simulink library "Garbage"  (R2023a)
settings: Solver=ode45  StartTime=0.0  StopTime=10.0  RelTol=1e-3  EnableMultiTasking=on
undefined symbols (not defined in the file or in its folder): c, ending_column_index, ending_row_index, framebuffer_16x1, framebuffer_2x2, framebuffer_4x4, framebuffer_8x8, HEIGHT, hot, POS_X, POS_Y, starting_column_index, starting_row_index, WIDTH
libraries: Memory_Blocks_Lib x459, simulink x235, simulink_extras x132, dspmtrx3 x116, Bit_Multiplexers_Lib x67, Bit_Demultiplexers_Lib x49, Byte_Multiplexers_Lib x40, Miscellaneous x38, Computer_Library x17, Decoders_Lib x14, commutil2 x12, Arithmetic_Operations_Lib x8
(part 5 of 26)

== system Garbage/Byte_Multiplexer_2_to_1 ==
  Addr(1b) : Inport
  Data_1(1B) : Inport  Port=2
  Data_0(1B) : Inport  Port=3
  Bit_Multiplexer_2_to_1(Bit_0) : Bit_Multiplexers_Lib/Bit_Multiplexers_Lib/Bit_Multiplexer_2_to_1  SystemSampleTime=-1  VariantActivationTime=update diagram  Latency=0  AutoFrameSizeCalculation=off
  Bit_Multiplexer_2_to_1(Bit_1) : Bit_Multiplexers_Lib/Bit_Multiplexers_Lib/Bit_Multiplexer_2_to_1  SystemSampleTime=-1  VariantActivationTime=update diagram  Latency=0  AutoFrameSizeCalculation=off
  Bit_Multiplexer_2_to_1(Bit_2) : Bit_Multiplexers_Lib/Bit_Multiplexers_Lib/Bit_Multiplexer_2_to_1  SystemSampleTime=-1  VariantActivationTime=update diagram  Latency=0  AutoFrameSizeCalculation=off
  Bit_Multiplexer_2_to_1(Bit_3) : Bit_Multiplexers_Lib/Bit_Multiplexers_Lib/Bit_Multiplexer_2_to_1  SystemSampleTime=-1  VariantActivationTime=update diagram  Latency=0  AutoFrameSizeCalculation=off
  Bit_Multiplexer_2_to_1(Bit_4) : Bit_Multiplexers_Lib/Bit_Multiplexers_Lib/Bit_Multiplexer_2_to_1  SystemSampleTime=-1  VariantActivationTime=update diagram  Latency=0  AutoFrameSizeCalculation=off
  Bit_Multiplexer_2_to_1(Bit_5) : Bit_Multiplexers_Lib/Bit_Multiplexers_Lib/Bit_Multiplexer_2_to_1  SystemSampleTime=-1  VariantActivationTime=update diagram  Latency=0  AutoFrameSizeCalculation=off
  Bit_Multiplexer_2_to_1(Bit_6) : Bit_Multiplexers_Lib/Bit_Multiplexers_Lib/Bit_Multiplexer_2_to_1  SystemSampleTime=-1  VariantActivationTime=update diagram  Latency=0  AutoFrameSizeCalculation=off
  Bit_Multiplexer_2_to_1(Bit_7) : Bit_Multiplexers_Lib/Bit_Multiplexers_Lib/Bit_Multiplexer_2_to_1  SystemSampleTime=-1  VariantActivationTime=update diagram  Latency=0  AutoFrameSizeCalculation=off
  Bus Creator : BusCreator  Inputs=8
  Bus Selector : BusSelector  OutputSignals=signal1,signal2,signal3,signal4,signal5,signal6,signal7,signal8
  Bus Selector1 : BusSelector  OutputSignals=signal1,signal2,signal3,signal4,signal5,signal6,signal7,signal8
  Data(1B) : Outport
  wires:
  Bus Creator/1 -> Data(1B)/1
  Addr(1b)/1 -> Bit_Multiplexer_2_to_1(Bit_7)/1, Bit_Multiplexer_2_to_1(Bit_0)/1, Bit_Multiplexer_2_to_1(Bit_1)/1, Bit_Multiplexer_2_to_1(Bit_2)/1, Bit_Multiplexer_2_to_1(Bit_3)/1, Bit_Multiplexer_2_to_1(Bit_4)/1, Bit_Multiplexer_2_to_1(Bit_5)/1, Bit_Multiplexer_2_to_1(Bit_6)/1
  Bit_Multiplexer_2_to_1(Bit_4)/1 -> Bus Creator/4
  Bit_Multiplexer_2_to_1(Bit_5)/1 -> Bus Creator/3
  Bit_Multiplexer_2_to_1(Bit_6)/1 -> Bus Creator/2
  Bit_Multiplexer_2_to_1(Bit_7)/1 -> Bus Creator/1
  Bit_Multiplexer_2_to_1(Bit_3)/1 -> Bus Creator/5
  Bit_Multiplexer_2_to_1(Bit_2)/1 -> Bus Creator/6
  Bit_Multiplexer_2_to_1(Bit_1)/1 -> Bus Creator/7
  Bit_Multiplexer_2_to_1(Bit_0)/1 -> Bus Creator/8
  Data_1(1B)/1 -> Bus Selector1/1
  Data_0(1B)/1 -> Bus Selector/1
  Bus Selector1/8 -> Bit_Multiplexer_2_to_1(Bit_0)/2  "<signal8>"
  Bus Selector/8 -> Bit_Multiplexer_2_to_1(Bit_0)/3  "<signal8>"
  Bus Selector1/7 -> Bit_Multiplexer_2_to_1(Bit_1)/2  "<signal7>"
  Bus Selector/7 -> Bit_Multiplexer_2_to_1(Bit_1)/3  "<signal7>"
  Bus Selector1/6 -> Bit_Multiplexer_2_to_1(Bit_2)/2  "<signal6>"
  Bus Selector/6 -> Bit_Multiplexer_2_to_1(Bit_2)/3  "<signal6>"
  Bus Selector/5 -> Bit_Multiplexer_2_to_1(Bit_3)/3  "<signal5>"
  Bus Selector1/5 -> Bit_Multiplexer_2_to_1(Bit_3)/2  "<signal5>"
  Bus Selector/4 -> Bit_Multiplexer_2_to_1(Bit_4)/3  "<signal4>"
  Bus Selector1/4 -> Bit_Multiplexer_2_to_1(Bit_4)/2  "<signal4>"
  Bus Selector1/3 -> Bit_Multiplexer_2_to_1(Bit_5)/2  "<signal3>"
  Bus Selector/3 -> Bit_Multiplexer_2_to_1(Bit_5)/3  "<signal3>"
  Bus Selector1/2 -> Bit_Multiplexer_2_to_1(Bit_6)/2  "<signal2>"
  Bus Selector/2 -> Bit_Multiplexer_2_to_1(Bit_6)/3  "<signal2>"
  Bus Selector/1 -> Bit_Multiplexer_2_to_1(Bit_7)/3  "<signal1>"
  Bus Selector1/1 -> Bit_Multiplexer_2_to_1(Bit_7)/2  "<signal1>"

== system Garbage/Byte_Multiplexer_4_to_1 ==
  Addr_1(1b) : Inport
  Addr_0(1b) : Inport  Port=2
  Data_3(1B) : Inport  Port=3
  Data_2(1B) : Inport  Port=4
  Data_1(1B) : Inport  Port=5
  Data_0(1B) : Inport  Port=6
  Bit_Multiplexer_4_to_1(Bit_0) : Bit_Multiplexers_Lib/Bit_Mux_4  SystemSampleTime=-1  VariantActivationTime=update diagram  Latency=0  AutoFrameSizeCalculation=off
  Bit_Multiplexer_4_to_1(Bit_1) : Bit_Multiplexers_Lib/Bit_Mux_4  SystemSampleTime=-1  VariantActivationTime=update diagram  Latency=0  AutoFrameSizeCalculation=off
  Bit_Multiplexer_4_to_1(Bit_2) : Bit_Multiplexers_Lib/Bit_Mux_4  SystemSampleTime=-1  VariantActivationTime=update diagram  Latency=0  AutoFrameSizeCalculation=off
  Bit_Multiplexer_4_to_1(Bit_3) : Bit_Multiplexers_Lib/Bit_Mux_4  SystemSampleTime=-1  VariantActivationTime=update diagram  Latency=0  AutoFrameSizeCalculation=off
  Bit_Multiplexer_4_to_1(Bit_4) : Bit_Multiplexers_Lib/Bit_Mux_4  SystemSampleTime=-1  VariantActivationTime=update diagram  Latency=0  AutoFrameSizeCalculation=off
  Bit_Multiplexer_4_to_1(Bit_5) : Bit_Multiplexers_Lib/Bit_Mux_4  SystemSampleTime=-1  VariantActivationTime=update diagram  Latency=0  AutoFrameSizeCalculation=off
  Bit_Multiplexer_4_to_1(Bit_6) : Bit_Multiplexers_Lib/Bit_Mux_4  SystemSampleTime=-1  VariantActivationTime=update diagram  Latency=0  AutoFrameSizeCalculation=off
  Bit_Multiplexer_4_to_1(Bit_7) : Bit_Multiplexers_Lib/Bit_Mux_4  SystemSampleTime=-1  VariantActivationTime=update diagram  Latency=0  AutoFrameSizeCalculation=off
  Bus Creator3 : BusCreator  Inputs=8
  Bus Selector{,1..3} (4 alike) : BusSelector  OutputSignals=signal1,signal2,signal3,signal4,signal5,signal6,signal7,signal8
  Data(1B) : Outport
  wires:
  Bus Creator3/1 -> Data(1B)/1
  Addr_0(1b)/1 -> Bit_Multiplexer_4_to_1(Bit_0)/2, Bit_Multiplexer_4_to_1(Bit_1)/2, Bit_Multiplexer_4_to_1(Bit_2)/2, Bit_Multiplexer_4_to_1(Bit_3)/2, Bit_Multiplexer_4_to_1(Bit_4)/2, Bit_Multiplexer_4_to_1(Bit_5)/2, Bit_Multiplexer_4_to_1(Bit_6)/2, Bit_Multiplexer_4_to_1(Bit_7)/2
  Addr_1(1b)/1 -> Bit_Multiplexer_4_to_1(Bit_7)/1, Bit_Multiplexer_4_to_1(Bit_0)/1, Bit_Multiplexer_4_to_1(Bit_1)/1, Bit_Multiplexer_4_to_1(Bit_2)/1, Bit_Multiplexer_4_to_1(Bit_3)/1, Bit_Multiplexer_4_to_1(Bit_4)/1, Bit_Multiplexer_4_to_1(Bit_5)/1, Bit_Multiplexer_4_to_1(Bit_6)/1
  Bit_Multiplexer_4_to_1(Bit_4)/1 -> Bus Creator3/4
  Bit_Multiplexer_4_to_1(Bit_5)/1 -> Bus Creator3/3
  Bit_Multiplexer_4_to_1(Bit_6)/1 -> Bus Creator3/2
  Bit_Multiplexer_4_to_1(Bit_7)/1 -> Bus Creator3/1
  Bit_Multiplexer_4_to_1(Bit_3)/1 -> Bus Creator3/5
  Bit_Multiplexer_4_to_1(Bit_2)/1 -> Bus Creator3/6
  Bit_Multiplexer_4_to_1(Bit_1)/1 -> Bus Creator3/7
  Bit_Multiplexer_4_to_1(Bit_0)/1 -> Bus Creator3/8
  Data_3(1B)/1 -> Bus Selector/1
  Data_2(1B)/1 -> Bus Selector1/1
  Data_1(1B)/1 -> Bus Selector2/1
  Data_0(1B)/1 -> Bus Selector3/1
  Bus Selector/8 -> Bit_Multiplexer_4_to_1(Bit_0)/3  "<signal8>"
  Bus Selector1/8 -> Bit_Multiplexer_4_to_1(Bit_0)/4  "<signal8>"
  Bus Selector2/8 -> Bit_Multiplexer_4_to_1(Bit_0)/5  "<signal8>"
  Bus Selector3/8 -> Bit_Multiplexer_4_to_1(Bit_0)/6  "<signal8>"
  Bus Selector/7 -> Bit_Multiplexer_4_to_1(Bit_1)/3  "<signal7>"
  Bus Selector1/7 -> Bit_Multiplexer_4_to_1(Bit_1)/4  "<signal7>"
  Bus Selector2/7 -> Bit_Multiplexer_4_to_1(Bit_1)/5  "<signal7>"
  Bus Selector3/7 -> Bit_Multiplexer_4_to_1(Bit_1)/6  "<signal7>"
  Bus Selector/6 -> Bit_Multiplexer_4_to_1(Bit_2)/3  "<signal6>"
  Bus Selector1/6 -> Bit_Multiplexer_4_to_1(Bit_2)/4  "<signal6>"
  Bus Selector2/6 -> Bit_Multiplexer_4_to_1(Bit_2)/5  "<signal6>"
  Bus Selector3/6 -> Bit_Multiplexer_4_to_1(Bit_2)/6  "<signal6>"
  Bus Selector/5 -> Bit_Multiplexer_4_to_1(Bit_3)/3  "<signal5>"
  Bus Selector1/5 -> Bit_Multiplexer_4_to_1(Bit_3)/4  "<signal5>"
  Bus Selector2/5 -> Bit_Multiplexer_4_to_1(Bit_3)/5  "<signal5>"
  Bus Selector3/5 -> Bit_Multiplexer_4_to_1(Bit_3)/6  "<signal5>"
  Bus Selector/4 -> Bit_Multiplexer_4_to_1(Bit_4)/3  "<signal4>"
  Bus Selector1/4 -> Bit_Multiplexer_4_to_1(Bit_4)/4  "<signal4>"
  Bus Selector2/4 -> Bit_Multiplexer_4_to_1(Bit_4)/5  "<signal4>"
  Bus Selector3/4 -> Bit_Multiplexer_4_to_1(Bit_4)/6  "<signal4>"
  Bus Selector/3 -> Bit_Multiplexer_4_to_1(Bit_5)/3  "<signal3>"
  Bus Selector1/3 -> Bit_Multiplexer_4_to_1(Bit_5)/4  "<signal3>"
  Bus Selector2/3 -> Bit_Multiplexer_4_to_1(Bit_5)/5  "<signal3>"
  Bus Selector3/3 -> Bit_Multiplexer_4_to_1(Bit_5)/6  "<signal3>"
  Bus Selector/2 -> Bit_Multiplexer_4_to_1(Bit_6)/3  "<signal2>"
  Bus Selector1/2 -> Bit_Multiplexer_4_to_1(Bit_6)/4  "<signal2>"
  Bus Selector2/2 -> Bit_Multiplexer_4_to_1(Bit_6)/5  "<signal2>"
  Bus Selector3/2 -> Bit_Multiplexer_4_to_1(Bit_6)/6  "<signal2>"
  Bus Selector/1 -> Bit_Multiplexer_4_to_1(Bit_7)/3  "<signal1>"
  Bus Selector1/1 -> Bit_Multiplexer_4_to_1(Bit_7)/4  "<signal1>"
  Bus Selector2/1 -> Bit_Multiplexer_4_to_1(Bit_7)/5  "<signal1>"
  Bus Selector3/1 -> Bit_Multiplexer_4_to_1(Bit_7)/6  "<signal1>"

== system Garbage/Byte_Multiplexer_8_to_1 ==
  Addr_2(1b) : Inport
  Addr_1(1b) : Inport  Port=2
  Addr_0(1b) : Inport  Port=3
  Data_7(1B) : Inport  Port=4
  Data_6(1B) : Inport  Port=5
  Data_5(1B) : Inport  Port=6
  Data_4(1B) : Inport  Port=7
  Data_3(1B) : Inport  Port=8
  Data_2(1B) : Inport  Port=9
  Data_1(1B) : Inport  Port=10
  Data_0(1B) : Inport  Port=11
  Bit_Multiplexer_8_to_1(Bit_0) : Bit_Multiplexers_Lib/Bit_MltPlx_8  SystemSampleTime=-1  VariantActivationTime=update diagram  Latency=0  AutoFrameSizeCalculation=off
  Bit_Multiplexer_8_to_1(Bit_1) : Bit_Multiplexers_Lib/Bit_MltPlx_8  SystemSampleTime=-1  VariantActivationTime=update diagram  Latency=0  AutoFrameSizeCalculation=off
  Bit_Multiplexer_8_to_1(Bit_2) : Bit_Multiplexers_Lib/Bit_MltPlx_8  SystemSampleTime=-1  VariantActivationTime=update diagram  Latency=0  AutoFrameSizeCalculation=off
  Bit_Multiplexer_8_to_1(Bit_3) : Bit_Multiplexers_Lib/Bit_MltPlx_8  SystemSampleTime=-1  VariantActivationTime=update diagram  Latency=0  AutoFrameSizeCalculation=off
  Bit_Multiplexer_8_to_1(Bit_4) : Bit_Multiplexers_Lib/Bit_MltPlx_8  SystemSampleTime=-1  VariantActivationTime=update diagram  Latency=0  AutoFrameSizeCalculation=off
  Bit_Multiplexer_8_to_1(Bit_5) : Bit_Multiplexers_Lib/Bit_MltPlx_8  SystemSampleTime=-1  VariantActivationTime=update diagram  Latency=0  AutoFrameSizeCalculation=off
  Bit_Multiplexer_8_to_1(Bit_6) : Bit_Multiplexers_Lib/Bit_MltPlx_8  SystemSampleTime=-1  VariantActivationTime=update diagram  Latency=0  AutoFrameSizeCalculation=off
  Bit_Multiplexer_8_to_1(Bit_7) : Bit_Multiplexers_Lib/Bit_MltPlx_8  SystemSampleTime=-1  VariantActivationTime=update diagram  Latency=0  AutoFrameSizeCalculation=off
  Bus Creator3 : BusCreator  Inputs=8
  Bus Selector{,1..7} (8 alike) : BusSelector  OutputSignals=signal1,signal2,signal3,signal4,signal5,signal6,signal7,signal8
  Data(1B) : Outport
  wires:
  Bus Creator3/1 -> Data(1B)/1
  Addr_0(1b)/1 -> Bit_Multiplexer_8_to_1(Bit_0)/3, Bit_Multiplexer_8_to_1(Bit_1)/3, Bit_Multiplexer_8_to_1(Bit_2)/3, Bit_Multiplexer_8_to_1(Bit_3)/3, Bit_Multiplexer_8_to_1(Bit_4)/3, Bit_Multiplexer_8_to_1(Bit_5)/3, Bit_Multiplexer_8_to_1(Bit_6)/3, Bit_Multiplexer_8_to_1(Bit_7)/3
  Addr_1(1b)/1 -> Bit_Multiplexer_8_to_1(Bit_5)/2, Bit_Multiplexer_8_to_1(Bit_0)/2, Bit_Multiplexer_8_to_1(Bit_1)/2, Bit_Multiplexer_8_to_1(Bit_2)/2, Bit_Multiplexer_8_to_1(Bit_3)/2, Bit_Multiplexer_8_to_1(Bit_4)/2, Bit_Multiplexer_8_to_1(Bit_6)/2, Bit_Multiplexer_8_to_1(Bit_7)/2
  Addr_2(1b)/1 -> Bit_Multiplexer_8_to_1(Bit_0)/1, Bit_Multiplexer_8_to_1(Bit_1)/1, Bit_Multiplexer_8_to_1(Bit_2)/1, Bit_Multiplexer_8_to_1(Bit_3)/1, Bit_Multiplexer_8_to_1(Bit_4)/1, Bit_Multiplexer_8_to_1(Bit_5)/1, Bit_Multiplexer_8_to_1(Bit_6)/1, Bit_Multiplexer_8_to_1(Bit_7)/1
  Bit_Multiplexer_8_to_1(Bit_4)/1 -> Bus Creator3/4
  Bit_Multiplexer_8_to_1(Bit_5)/1 -> Bus Creator3/3
  Bit_Multiplexer_8_to_1(Bit_6)/1 -> Bus Creator3/2
  Bit_Multiplexer_8_to_1(Bit_7)/1 -> Bus Creator3/1
  Bit_Multiplexer_8_to_1(Bit_3)/1 -> Bus Creator3/5
  Bit_Multiplexer_8_to_1(Bit_2)/1 -> Bus Creator3/6
  Bit_Multiplexer_8_to_1(Bit_1)/1 -> Bus Creator3/7
  Bit_Multiplexer_8_to_1(Bit_0)/1 -> Bus Creator3/8
  Data_7(1B)/1 -> Bus Selector/1
  Data_6(1B)/1 -> Bus Selector1/1
  Data_5(1B)/1 -> Bus Selector2/1
  Data_4(1B)/1 -> Bus Selector3/1
  Data_3(1B)/1 -> Bus Selector4/1
  Data_2(1B)/1 -> Bus Selector5/1
  Data_1(1B)/1 -> Bus Selector6/1
  Data_0(1B)/1 -> Bus Selector7/1
  Bus Selector/1 -> Bit_Multiplexer_8_to_1(Bit_7)/4  "<signal1>"
  Bus Selector1/1 -> Bit_Multiplexer_8_to_1(Bit_7)/5  "<signal1>"
  Bus Selector2/1 -> Bit_Multiplexer_8_to_1(Bit_7)/6  "<signal1>"
  Bus Selector3/1 -> Bit_Multiplexer_8_to_1(Bit_7)/7  "<signal1>"
  Bus Selector4/1 -> Bit_Multiplexer_8_to_1(Bit_7)/8  "<signal1>"
  Bus Selector5/1 -> Bit_Multiplexer_8_to_1(Bit_7)/9  "<signal1>"
  Bus Selector6/1 -> Bit_Multiplexer_8_to_1(Bit_7)/10  "<signal1>"
  Bus Selector7/1 -> Bit_Multiplexer_8_to_1(Bit_7)/11  "<signal1>"
  Bus Selector/2 -> Bit_Multiplexer_8_to_1(Bit_6)/4  "<signal2>"
  Bus Selector1/2 -> Bit_Multiplexer_8_to_1(Bit_6)/5  "<signal2>"
  Bus Selector2/2 -> Bit_Multiplexer_8_to_1(Bit_6)/6  "<signal2>"
  Bus Selector3/2 -> Bit_Multiplexer_8_to_1(Bit_6)/7  "<signal2>"
  Bus Selector4/2 -> Bit_Multiplexer_8_to_1(Bit_6)/8  "<signal2>"
  Bus Selector5/2 -> Bit_Multiplexer_8_to_1(Bit_6)/9  "<signal2>"
  Bus Selector6/2 -> Bit_Multiplexer_8_to_1(Bit_6)/10  "<signal2>"
  Bus Selector7/2 -> Bit_Multiplexer_8_to_1(Bit_6)/11  "<signal2>"
  Bus Selector/3 -> Bit_Multiplexer_8_to_1(Bit_5)/4  "<signal3>"
  Bus Selector1/3 -> Bit_Multiplexer_8_to_1(Bit_5)/5  "<signal3>"
  Bus Selector2/3 -> Bit_Multiplexer_8_to_1(Bit_5)/6  "<signal3>"
  Bus Selector3/3 -> Bit_Multiplexer_8_to_1(Bit_5)/7  "<signal3>"
  Bus Selector4/3 -> Bit_Multiplexer_8_to_1(Bit_5)/8  "<signal3>"
  Bus Selector5/3 -> Bit_Multiplexer_8_to_1(Bit_5)/9  "<signal3>"
  Bus Selector6/3 -> Bit_Multiplexer_8_to_1(Bit_5)/10  "<signal3>"
  Bus Selector7/3 -> Bit_Multiplexer_8_to_1(Bit_5)/11  "<signal3>"
  Bus Selector/4 -> Bit_Multiplexer_8_to_1(Bit_4)/4  "<signal4>"
  Bus Selector1/4 -> Bit_Multiplexer_8_to_1(Bit_4)/5  "<signal4>"
  Bus Selector2/4 -> Bit_Multiplexer_8_to_1(Bit_4)/6  "<signal4>"
  Bus Selector3/4 -> Bit_Multiplexer_8_to_1(Bit_4)/7  "<signal4>"
  Bus Selector4/4 -> Bit_Multiplexer_8_to_1(Bit_4)/8  "<signal4>"
  Bus Selector5/4 -> Bit_Multiplexer_8_to_1(Bit_4)/9  "<signal4>"
  Bus Selector6/4 -> Bit_Multiplexer_8_to_1(Bit_4)/10  "<signal4>"
  Bus Selector7/4 -> Bit_Multiplexer_8_to_1(Bit_4)/11  "<signal4>"
  Bus Selector/5 -> Bit_Multiplexer_8_to_1(Bit_3)/4  "<signal5>"
  Bus Selector1/5 -> Bit_Multiplexer_8_to_1(Bit_3)/5  "<signal5>"
  Bus Selector2/5 -> Bit_Multiplexer_8_to_1(Bit_3)/6  "<signal5>"
  Bus Selector3/5 -> Bit_Multiplexer_8_to_1(Bit_3)/7  "<signal5>"
  Bus Selector4/5 -> Bit_Multiplexer_8_to_1(Bit_3)/8  "<signal5>"
  Bus Selector5/5 -> Bit_Multiplexer_8_to_1(Bit_3)/9  "<signal5>"
  Bus Selector6/5 -> Bit_Multiplexer_8_to_1(Bit_3)/10  "<signal5>"
  Bus Selector7/5 -> Bit_Multiplexer_8_to_1(Bit_3)/11  "<signal5>"
  Bus Selector/6 -> Bit_Multiplexer_8_to_1(Bit_2)/4  "<signal6>"
  Bus Selector1/6 -> Bit_Multiplexer_8_to_1(Bit_2)/5  "<signal6>"
  Bus Selector2/6 -> Bit_Multiplexer_8_to_1(Bit_2)/6  "<signal6>"
  Bus Selector3/6 -> Bit_Multiplexer_8_to_1(Bit_2)/7  "<signal6>"
  Bus Selector4/6 -> Bit_Multiplexer_8_to_1(Bit_2)/8  "<signal6>"
  Bus Selector5/6 -> Bit_Multiplexer_8_to_1(Bit_2)/9  "<signal6>"
  Bus Selector6/6 -> Bit_Multiplexer_8_to_1(Bit_2)/10  "<signal6>"
  Bus Selector7/6 -> Bit_Multiplexer_8_to_1(Bit_2)/11  "<signal6>"
  Bus Selector/7 -> Bit_Multiplexer_8_to_1(Bit_1)/4  "<signal7>"
  Bus Selector1/7 -> Bit_Multiplexer_8_to_1(Bit_1)/5  "<signal7>"
  Bus Selector2/7 -> Bit_Multiplexer_8_to_1(Bit_1)/6  "<signal7>"
  Bus Selector3/7 -> Bit_Multiplexer_8_to_1(Bit_1)/7  "<signal7>"
  Bus Selector4/7 -> Bit_Multiplexer_8_to_1(Bit_1)/8  "<signal7>"
  Bus Selector5/7 -> Bit_Multiplexer_8_to_1(Bit_1)/9  "<signal7>"
  Bus Selector6/7 -> Bit_Multiplexer_8_to_1(Bit_1)/10  "<signal7>"
  Bus Selector7/7 -> Bit_Multiplexer_8_to_1(Bit_1)/11  "<signal7>"
  Bus Selector/8 -> Bit_Multiplexer_8_to_1(Bit_0)/4  "<signal8>"
  Bus Selector1/8 -> Bit_Multiplexer_8_to_1(Bit_0)/5  "<signal8>"
  Bus Selector2/8 -> Bit_Multiplexer_8_to_1(Bit_0)/6  "<signal8>"
  Bus Selector3/8 -> Bit_Multiplexer_8_to_1(Bit_0)/7  "<signal8>"
  Bus Selector4/8 -> Bit_Multiplexer_8_to_1(Bit_0)/8  "<signal8>"
  Bus Selector5/8 -> Bit_Multiplexer_8_to_1(Bit_0)/9  "<signal8>"
  Bus Selector6/8 -> Bit_Multiplexer_8_to_1(Bit_0)/10  "<signal8>"
  Bus Selector7/8 -> Bit_Multiplexer_8_to_1(Bit_0)/11  "<signal8>"

== system Garbage/Counter_15bit ==
  CLK : Inport
  Bit_00 : simulink_extras/Flip Flops/J-K Flip-Flop  initial_condition=0
  Bit_01 : simulink_extras/Flip Flops/J-K Flip-Flop  initial_condition=0
  Bit_02 : simulink_extras/Flip Flops/J-K Flip-Flop  initial_condition=0
  Bit_03 : simulink_extras/Flip Flops/J-K Flip-Flop  initial_condition=0
  Bit_04 : simulink_extras/Flip Flops/J-K Flip-Flop  initial_condition=0
  Bit_05 : simulink_extras/Flip Flops/J-K Flip-Flop  initial_condition=0
  Bit_06 : simulink_extras/Flip Flops/J-K Flip-Flop  initial_condition=0
  Bit_07 : simulink_extras/Flip Flops/J-K Flip-Flop  initial_condition=0
  Bit_08 : simulink_extras/Flip Flops/J-K Flip-Flop  initial_condition=0
  Bit_09 : simulink_extras/Flip Flops/J-K Flip-Flop  initial_condition=0
  Bit_{10..14} (5 alike) : simulink_extras/Flip Flops/J-K Flip-Flop  initial_condition=0
  Increment Enabled1 : Logic  IconShape=distinctive  AllPortsSameDT=off  OutDataTypeStr=boolean
  Increment Enabled2 : Logic  IconShape=distinctive  AllPortsSameDT=off  OutDataTypeStr=boolean
  Increment(1b) : Constant  OutDataTypeStr=boolean
  Logical Operator{1..10,13} (11 alike) : Logic  IconShape=distinctive  AllPortsSameDT=off  OutDataTypeStr=boolean
  Logical Operator11 : Logic  Inputs=15  IconShape=distinctive  AllPortsSameDT=off  OutDataTypeStr=boolean
  Terminator{1..15} (15 alike) : Terminator
  1Hz : Outport
  wires:
  Bit_00/2 -> Terminator9/1
  Increment(1b)/1 -> Bit_00/3, Bit_00/1
  Bit_02/2 -> Terminator11/1
  Bit_03/2 -> Terminator12/1
  Bit_01/2 -> Terminator10/1
  Logical Operator2/1 -> Logical Operator10/1, Bit_07/1, Bit_07/3
  Bit_07/1 -> Logical Operator11/8, Logical Operator10/2
  Bit_06/2 -> Terminator2/1
  Bit_05/2 -> Terminator1/1
  Increment Enabled1/1 -> Bit_05/3, Bit_05/1, Logical Operator1/1
  Bit_07/2 -> Terminator3/1
  Logical Operator13/1 -> Increment Enabled1/1, Bit_04/3, Bit_04/1
  Logical Operator1/1 -> Logical Operator2/1, Bit_06/1, Bit_06/3
  Logical Operator4/1 -> Logical Operator13/1, Bit_03/3, Bit_03/1
  Bit_04/2 -> Terminator4/1
  Logical Operator3/1 -> Logical Operator4/1, Bit_02/3, Bit_02/1
  Bit_09/1 -> Logical Operator11/10, Logical Operator9/2
  Logical Operator10/1 -> Bit_08/1, Bit_08/3, Logical Operator8/1
  Bit_10/1 -> Logical Operator11/11, Logical Operator6/2
  Bit_09/2 -> Terminator7/1
  Bit_10/2 -> Terminator8/1
  Bit_08/2 -> Terminator6/1
  Bit_08/1 -> Logical Operator11/9, Logical Operator8/2
  Bit_13/1 -> Logical Operator11/14, Logical Operator7/2
  Bit_11/1 -> Logical Operator11/12, Increment Enabled2/2
  Bit_12/1 -> Logical Operator11/13, Logical Operator5/2
  Logical Operator7/1 -> Bit_14/1, Bit_14/3
  CLK/1 -> Bit_00/2, Bit_01/2, Bit_02/2, Bit_03/2, Bit_04/2, Bit_05/2, Bit_06/2, Bit_07/2, Bit_08/2, Bit_09/2, Bit_10/2, Bit_11/2, Bit_12/2, Bit_13/2, Bit_14/2
  Bit_14/1 -> Logical Operator11/15
  Bit_13/2 -> Terminator13/1
  Bit_12/2 -> Terminator5/1
  Increment Enabled2/1 -> Bit_12/3, Bit_12/1, Logical Operator5/1
  Bit_14/2 -> Terminator14/1
  Logical Operator6/1 -> Increment Enabled2/1, Bit_11/3, Bit_11/1
  Logical Operator5/1 -> Logical Operator7/1, Bit_13/1, Bit_13/3
  Logical Operator9/1 -> Logical Operator6/1, Bit_10/3, Bit_10/1
  Bit_11/2 -> Terminator15/1
  Logical Operator8/1 -> Logical Operator9/1, Bit_09/3, Bit_09/1
  Bit_06/1 -> Logical Operator11/7, Logical Operator2/2
  Bit_05/1 -> Logical Operator11/6, Logical Operator1/2
  Bit_04/1 -> Logical Operator11/5, Increment Enabled1/2
  Bit_03/1 -> Logical Operator11/4, Logical Operator13/2
  Bit_02/1 -> Logical Operator11/3, Logical Operator4/2
  Bit_01/1 -> Logical Operator11/2, Logical Operator3/2
  Bit_00/1 -> Bit_01/1, Bit_01/3, Logical Operator11/1, Logical Operator3/1
  Logical Operator11/1 -> 1Hz/1

== system Garbage/Counter_8bit_Cfg ==
  note: Bit_MltPlx_2_to_1
  note: Bit_MltPlx_2_to_1
  note: Bit_MltPlx_2_to_1
  note: Bit_MltPlx_2_to_1
  note: Bit_MltPlx_2_to_1
  note: Bit_MltPlx_2_to_1
  note: Bit_MltPlx_2_to_1
  note: Bit_MltPlx_2_to_1
  note: Bit_MltPlx_2_to_1
  note: Bit_MltPlx_2_to_1
  note: Bit_MltPlx_2_to_1
  note: Bit_MltPlx_2_to_1
  note: Bit_MltPlx_2_to_1
  note: Bit_MltPlx_2_to_1
  note: Bit_MltPlx_2_to_1
  note: Bit_MltPlx_2_to_1
  Trshld(1B) : Inport
  SetDirect(1b) : Inport  Port=2
  ValIn(1B) : Inport  Port=3
  CLK : Inport  Port=4
  Bit_{0..7} (8 alike) : simulink_extras/Flip Flops/J-K Flip-Flop  initial_condition=0
  Bus Creator : BusCreator  Inputs=8
  Bus Selector : BusSelector  OutputSignals=signal1,signal2,signal3,signal4,signal5,signal6,signal7,signal8
  Bus Selector4 : BusSelector  OutputSignals=signal1,signal2,signal3,signal4,signal5,signal6,signal7,signal8
  Increment Enabled(00) : Logic  IconShape=distinctive  AllPortsSameDT=off  OutDataTypeStr=boolean
  Increment Enabled(01) : Logic  IconShape=distinctive  AllPortsSameDT=off  OutDataTypeStr=boolean
  Increment Enabled(02) : Logic  IconShape=distinctive  AllPortsSameDT=off  OutDataTypeStr=boolean
  Increment Enabled(03) : Logic  IconShape=distinctive  AllPortsSameDT=off  OutDataTypeStr=boolean
  Increment Enabled(04) : Logic  IconShape=distinctive  AllPortsSameDT=off  OutDataTypeStr=boolean
  Increment Enabled(05) : Logic  IconShape=distinctive  AllPortsSameDT=off  OutDataTypeStr=boolean
  Increment Enabled(06) : Logic  IconShape=distinctive  AllPortsSameDT=off  OutDataTypeStr=boolean
  Logical Operator{,32..34,36,71,82,93,117,128} (10 alike) : Logic  Operator=NOT  IconShape=distinctive  AllPortsSameDT=off  OutDataTypeStr=boolean
  Logical Operator{35,38..39,41..42,45..46,49..51,54..55,58..59,63..64,67..68,72..73,76..77,80..81,85..86,89..90,94..95,98..105,115..116} (40 alike) : Logic  IconShape=distinctive  AllPortsSameDT=off  OutDataTypeStr=boolean
  Logical Operator{5..7,11,13,18,20,30} (8 alike) : Logic  Operator=NXOR  IconShape=distinctive  AllPortsSameDT=off  OutDataTypeStr=boolean
  Logical Operator{43,47,52,56,60..61,65,69,74,78,83,87,91,96,113,118} (16 alike) : Logic  Operator=OR  IconShape=distinctive  OutDataTypeStr=boolean
  Logical Operator{31,40,44,48,53,57,62,66,70,75,79,84,88,92,97,114} (16 alike) : Logic  Operator=NOT  Inputs=4  IconShape=distinctive  AllPortsSameDT=off  OutDataTypeStr=boolean
  Logical Operator37 : Logic  Operator=OR  IconShape=distinctive  AllPortsSameDT=off  OutDataTypeStr=boolean
  "Reset " : Logic  Inputs=8  IconShape=distinctive  AllPortsSameDT=off  OutDataTypeStr=boolean
  Terminator{5..8,17..19,24} (8 alike) : Terminator
  RstOut(1b) : Outport
  ValOut(1B) : Outport  Port=2
  wires:
  Bit_3/2 -> Terminator8/1
  Logical Operator40/1 -> Logical Operator42/1
  Logical Operator38/1 -> Logical Operator60/1
  Logical Operator49/1 -> Logical Operator60/2
  Logical Operator31/1 -> Logical Operator49/1
  CLK/1 -> Bit_0/2, Bit_1/2, Bit_2/2, Bit_3/2, Bit_4/2, Bit_5/2, Bit_6/2, Bit_7/2
  Logical Operator69/1 -> Bit_3/3
  Logical Operator33/1 -> Logical Operator94/2
  Increment Enabled(00)/1 -> Increment Enabled(01)/1, Logical Operator51/2, Logical Operator46/2
  Bit_0/2 -> Terminator24/1
  Logical Operator39/1 -> Logical Operator50/2, Logical Operator117/1
  Logical Operator60/1 -> Bit_0/1
  ValIn(1B)/1 -> Bus Selector/1
  Logical Operator61/1 -> Bit_2/3
  Bit_4/2 -> Terminator19/1
  Logical Operator71/1 -> Logical Operator67/2
  Logical Operator65/1 -> Bit_3/1
  Logical Operator87/1 -> Bit_5/3
  Logical Operator78/1 -> Bit_4/3
  Bit_6/2 -> Terminator17/1
  Bit_7/2 -> Terminator18/1
  Increment Enabled(06)/1 -> Logical Operator116/2, Logical Operator99/2
  Logical Operator117/1 -> Logical Operator45/2
  Logical Operator104/1 -> Logical Operator33/1, Logical Operator89/2
  Logical Operator32/1 -> Logical Operator85/2
  Logical Operator75/1 -> Logical Operator77/1
  Logical Operator77/1 -> Logical Operator78/2
  Logical Operator63/1 -> Logical Operator65/1
  Logical Operator45/1 -> Logical Operator47/1
  Logical Operator59/1 -> Logical Operator61/2
  Bus Creator/1 -> ValOut(1B)/1
  Logical Operator64/1 -> Logical Operator65/2
  Logical Operator46/1 -> Logical Operator47/2
  Logical Operator99/1 -> Logical Operator113/2
  Logical Operator53/1 -> Logical Operator55/1
  Logical Operator72/1 -> Logical Operator74/1
  Logical Operator74/1 -> Bit_4/1
  Increment Enabled(03)/1 -> Increment Enabled(04)/1, Logical Operator77/2, Logical Operator73/2
  Logical Operator116/1 -> Logical Operator118/2
  Logical Operator68/1 -> Logical Operator69/2
  Bit_5/2 -> Terminator5/1
  Logical Operator52/1 -> Bit_1/1
  Logical Operator44/1 -> Logical Operator46/1
  Logical Operator97/1 -> Logical Operator99/1
  Increment Enabled(01)/1 -> Increment Enabled(02)/1, Logical Operator55/2, Logical Operator59/2
  Logical Operator42/1 -> Logical Operator43/2
  Increment Enabled(02)/1 -> Increment Enabled(03)/1, Logical Operator68/2, Logical Operator64/2
  Logical Operator89/1 -> Logical Operator91/1
  Logical Operator66/1 -> Logical Operator68/1
  Logical Operator57/1 -> Logical Operator59/1
  Logical Operator50/1 -> Logical Operator52/1
  Logical Operator95/1 -> Logical Operator96/2
  Logical Operator88/1 -> Logical Operator90/1
  Logical Operator92/1 -> Logical Operator95/1
  Logical Operator41/1 -> Logical Operator43/1
  Logical Operator91/1 -> Bit_6/1
  Logical Operator56/1 -> Bit_2/1
  Logical Operator/1 -> Increment Enabled(00)/1, Logical Operator49/2, Logical Operator42/2
  Logical Operator73/1 -> Logical Operator74/2
  Logical Operator58/1 -> Logical Operator61/1
  Logical Operator128/1 -> Logical Operator58/2
  Logical Operator85/1 -> Logical Operator87/1
  Logical Operator84/1 -> Logical Operator86/1
  Logical Operator55/1 -> Logical Operator56/2
  Logical Operator51/1 -> Logical Operator52/2
  Logical Operator67/1 -> Logical Operator69/1
  Logical Operator101/1 -> Logical Operator63/2, Logical Operator71/1
  Logical Operator81/1 -> Logical Operator83/2
  Logical Operator80/1 -> Logical Operator83/1
  Logical Operator114/1 -> Logical Operator116/1
  Logical Operator100/1 -> Logical Operator128/1, Logical Operator54/2
  Logical Operator113/1 -> Bit_7/1
  Increment Enabled(05)/1 -> Logical Operator90/2, Logical Operator95/2, Increment Enabled(06)/1
  Logical Operator86/1 -> Logical Operator87/2
  Logical Operator62/1 -> Logical Operator64/1
  Logical Operator83/1 -> Bit_5/1
  Logical Operator37/1 -> Logical Operator/1, Logical Operator31/1, Logical Operator38/1, Logical Operator40/1, Logical Operator41/1, Logical Operator97/1, Logical Operator98/1, Logical Operator114/1, Logical Operator115/1, Logical Operator88/1, Logical Operator89/1, Logical Operator92/1, Logical Operator94/1, Logical Operator79/1, Logical Operator80/1, Logical Operator84/1, Logical Operator85/1, Logical Operator70/1, Logical Operator72/1, Logical Operator75/1, Logical Operator76/1, Logical Operator66/1, Logical Operator67/1, Logical Operator62/1, Logical Operator63/1, Logical Operator53/1, Logical Operator54/1, Logical Operator57/1, Logical Operator58/1, Logical Operator44/1, Logical Operator45/1, Logical Operator48/1, Logical Operator50/1
  Logical Operator96/1 -> Bit_6/3
  Logical Operator48/1 -> Logical Operator51/1
  Logical Operator70/1 -> Logical Operator73/1
  Logical Operator35/1 -> Logical Operator82/1, Logical Operator38/2
  Logical Operator34/1 -> Logical Operator76/2
  Logical Operator93/1 -> Logical Operator115/2
  Bit_2/2 -> Terminator7/1
  Logical Operator105/1 -> Logical Operator98/2, Logical Operator93/1
  Logical Operator98/1 -> Logical Operator113/1
  Bit_1/2 -> Terminator6/1
  Logical Operator102/1 -> Logical Operator72/2, Logical Operator34/1
  Logical Operator54/1 -> Logical Operator56/1
  Logical Operator103/1 -> Logical Operator80/2, Logical Operator32/1
  Logical Operator94/1 -> Logical Operator96/1
  Logical Operator90/1 -> Logical Operator91/2
  Logical Operator43/1 -> Bit_0/3
  Logical Operator47/1 -> Bit_1/3
  Logical Operator76/1 -> Logical Operator78/1
  Increment Enabled(04)/1 -> Increment Enabled(05)/1, Logical Operator86/2, Logical Operator81/2
  Logical Operator115/1 -> Logical Operator118/1
  Logical Operator79/1 -> Logical Operator81/1
  Logical Operator82/1 -> Logical Operator41/2
  Logical Operator118/1 -> Bit_7/3
  Bus Selector4/6 -> Logical Operator6/1  "<signal6>"
  Logical Operator5/1 -> "Reset "/5
  Bus Selector4/5 -> Logical Operator5/1  "<signal5>"
  Logical Operator30/1 -> "Reset "/8
  Bus Selector4/7 -> Logical Operator7/1  "<signal7>"
  Bus Selector4/8 -> Logical Operator30/1  "<signal8>"
  Logical Operator20/1 -> "Reset "/4
  Trshld(1B)/1 -> Bus Selector4/1
  Logical Operator7/1 -> "Reset "/7
  Bit_3/1 -> Logical Operator5/2, Bus Creator/5, Increment Enabled(03)/2
  Logical Operator6/1 -> "Reset "/6
  Bit_7/1 -> Logical Operator11/2, Bus Creator/1
  Bus Selector4/4 -> Logical Operator20/1  "<signal4>"
  "Reset "/1 -> Logical Operator36/1, Logical Operator37/1, RstOut(1b)/1
  Bit_5/1 -> Logical Operator18/2, Bus Creator/3, Increment Enabled(05)/2
  Bus Selector4/3 -> Logical Operator18/1  "<signal3>"
  Logical Operator13/1 -> "Reset "/2
  Bit_4/1 -> Logical Operator20/2, Bus Creator/4, Increment Enabled(04)/2
  Bit_0/1 -> Increment Enabled(00)/2, Logical Operator30/2, Bus Creator/8
  Bus Selector4/2 -> Logical Operator13/1  "<signal2>"
  Logical Operator18/1 -> "Reset "/3
  Bit_2/1 -> Logical Operator6/2, Bus Creator/6, Increment Enabled(02)/2
  Logical Operator11/1 -> "Reset "/1
  Bit_1/1 -> Logical Operator7/2, Bus Creator/7, Increment Enabled(01)/2
  Bit_6/1 -> Logical Operator13/2, Bus Creator/2, Increment Enabled(06)/2
  Bus Selector4/1 -> Logical Operator11/1  "<signal1>"
  Bus Selector/8 -> Logical Operator35/1  "<signal8>"
  SetDirect(1b)/1 -> Logical Operator37/2
  Bus Selector/7 -> Logical Operator39/1  "<signal7>"
  Bus Selector/6 -> Logical Operator100/1  "<signal6>"
  Bus Selector/5 -> Logical Operator101/1  "<signal5>"
  Bus Selector/4 -> Logical Operator102/1  "<signal4>"
  Bus Selector/3 -> Logical Operator103/1  "<signal3>"
  Bus Selector/2 -> Logical Operator104/1  "<signal2>"
  Bus Selector/1 -> Logical Operator105/1  "<signal1>"
  Logical Operator36/1 -> Logical Operator105/2, Logical Operator104/2, Logical Operator103/2, Logical Operator102/2, Logical Operator101/2, Logical Operator39/2, Logical Operator35/2, Logical Operator100/2

== system Garbage/Counter_8bit_Cfg__ ==
  Reset(1b) : Inport
  Trshld(1B) : Inport  Port=2
  CLK : Inport  Port=3
  Bit_{0..7} (8 alike) : simulink_extras/Flip Flops/J-K Flip-Flop  initial_condition=0
  Bus Creator11 : BusCreator  Inputs=8
  Bus Selector4 : BusSelector  OutputSignals=signal1,signal2,signal3,signal4,signal5,signal6,signal7,signal8
  Increment(1b) : Constant  OutDataTypeStr=boolean
  Logical Operator{1,3,9,14,19,22,25,28} (8 alike) : Logic  Operator=OR  IconShape=distinctive  AllPortsSameDT=off  OutDataTypeStr=boolean
  Logical Operator{2,4,8,10,15..17,21,23..24,26..27,29} (13 alike) : Logic  IconShape=distinctive  AllPortsSameDT=off  OutDataTypeStr=boolean
  Logical Operator{5..7,11,13,18,20,30} (8 alike) : Logic  Operator=NXOR  IconShape=distinctive  AllPortsSameDT=off  OutDataTypeStr=boolean
  Logical Operator12 : Logic  Operator=NOT  IconShape=distinctive  AllPortsSameDT=off  OutDataTypeStr=boolean
  "Reset " : Logic  Inputs=8  IconShape=distinctive  AllPortsSameDT=off  OutDataTypeStr=boolean
  Terminator{1..4,9..12} (8 alike) : Terminator
  RstOut(1b) : Outport
  Value(1B) : Outport  Port=2
  wires:
  Bit_0/2 -> Terminator9/1
  Bit_2/2 -> Terminator11/1
  Bit_3/2 -> Terminator12/1
  Bit_1/2 -> Terminator10/1
  Logical Operator17/1 -> Logical Operator22/1, Logical Operator23/1, Logical Operator2/1
  Logical Operator16/1 -> Logical Operator17/1, Logical Operator21/1, Logical Operator19/1
  Bit_2/1 -> Logical Operator17/2, Bus Creator11/6, Logical Operator6/2
  Bit_1/1 -> Logical Operator16/2, Bus Creator11/7, Logical Operator7/2
  Bit_0/1 -> Logical Operator16/1, Logical Operator14/1, Logical Operator15/1, Bus Creator11/8, Logical Operator30/2
  Bus Selector4/1 -> Logical Operator11/1  "<signal1>"
  Bus Selector4/2 -> Logical Operator13/1  "<signal2>"
  Bus Selector4/3 -> Logical Operator18/1  "<signal3>"
  Bus Selector4/4 -> Logical Operator20/1  "<signal4>"
  Logical Operator11/1 -> "Reset "/1
  Logical Operator13/1 -> "Reset "/2
  Logical Operator18/1 -> "Reset "/3
  Logical Operator20/1 -> "Reset "/4
  Increment(1b)/1 -> Bit_0/3
  Logical Operator14/1 -> Bit_1/3
  Logical Operator15/1 -> Bit_1/1
  Logical Operator21/1 -> Bit_2/1
  Logical Operator19/1 -> Bit_2/3
  Logical Operator23/1 -> Bit_3/1
  Logical Operator22/1 -> Bit_3/3
  "Reset "/1 -> RstOut(1b)/1, Logical Operator1/1
  Reset(1b)/1 -> Logical Operator1/2
  Bus Creator11/1 -> Value(1B)/1
  Trshld(1B)/1 -> Bus Selector4/1
  Logical Operator2/1 -> Logical Operator8/1, Logical Operator3/1, Logical Operator4/1
  Logical Operator3/1 -> Bit_4/3
  Logical Operator4/1 -> Bit_4/1
  Bit_3/1 -> Logical Operator2/2, Bus Creator11/5, Logical Operator5/2
  Logical Operator10/1 -> Bit_5/1
  Logical Operator8/1 -> Logical Operator9/1, Logical Operator10/1, Logical Operator29/1
  Logical Operator9/1 -> Bit_5/3
  Bit_4/1 -> Bus Creator11/4, Logical Operator20/2, Logical Operator8/2
  Bit_4/2 -> Terminator1/1
  CLK/1 -> Bit_0/2, Bit_2/2, Bit_3/2, Bit_4/2, Bit_7/2, Bit_6/2, Bit_5/2, Bit_1/2
  Bit_6/2 -> Terminator2/1
  Logical Operator26/1 -> Bit_6/1
  Bit_6/1 -> Bus Creator11/2, Logical Operator13/2, Logical Operator27/2
  Logical Operator27/1 -> Logical Operator28/1, Logical Operator24/1
  Logical Operator25/1 -> Bit_6/3
  Logical Operator24/1 -> Bit_7/1
  Logical Operator1/1 -> Logical Operator14/2, Logical Operator25/2, Logical Operator28/2, Logical Operator9/2, Logical Operator3/2, Logical Operator22/2, Logical Operator19/2, Logical Operator12/1
  Logical Operator28/1 -> Bit_7/3
  Logical Operator29/1 -> Logical Operator27/1, Logical Operator25/1, Logical Operator26/1
  Logical Operator12/1 -> Logical Operator24/2, Logical Operator26/2, Logical Operator10/2, Logical Operator4/2, Logical Operator23/2, Logical Operator21/2, Logical Operator15/2, Bit_0/1
  Bit_5/1 -> Bus Creator11/3, Logical Operator18/2, Logical Operator29/2
  Bit_5/2 -> Terminator3/1
  Bit_7/2 -> Terminator4/1
  Bit_7/1 -> Bus Creator11/1, Logical Operator11/2
  Logical Operator5/1 -> "Reset "/5
  Logical Operator6/1 -> "Reset "/6
  Logical Operator7/1 -> "Reset "/7
  Logical Operator30/1 -> "Reset "/8
  Bus Selector4/5 -> Logical Operator5/1  "<signal5>"
  Bus Selector4/6 -> Logical Operator6/1  "<signal6>"
  Bus Selector4/7 -> Logical Operator7/1  "<signal7>"
  Bus Selector4/8 -> Logical Operator30/1  "<signal8>"

== system Garbage/Dec_16 ==
  note: Decoder_4_to_16
  Addr_3(1b) : Inport
  Addr_2(1b) : Inport  Port=2
  Addr_1(1b) : Inport  Port=3
  Addr_0(1b) : Inport  Port=4
  Lo`gical Operator4 : Logic  Operator=NOT  IconShape=distinctive  AllPortsSameDT=off  OutDataTypeStr=boolean
  Logical Operator{,1..4,6..16} (16 alike) : Logic  Inputs=4  IconShape=distinctive  AllPortsSameDT=off  OutDataTypeStr=boolean
  Logical Operator22 : Logic  Operator=NOT  IconShape=distinctive  AllPortsSameDT=off  OutDataTypeStr=boolean
  Logical Operator23 : Logic  Operator=NOT  IconShape=distinctive  AllPortsSameDT=off  OutDataTypeStr=boolean
  Logical Operator5 : Logic  Operator=NOT  IconShape=distinctive  AllPortsSameDT=off  OutDataTypeStr=boolean
  Select_15(1b) : Outport
  Select_14(1b) : Outport  Port=2
  Select_13(1b) : Outport  Port=3
  Select_12(1b) : Outport  Port=4
  Select_11(1b) : Outport  Port=5
  Select_10(1b) : Outport  Port=6
  Select_09(1b) : Outport  Port=7
  Select_08(1b) : Outport  Port=8
  Select_07(1b) : Outport  Port=9
  Select_06(1b) : Outport  Port=10
  Select_05(1b) : Outport  Port=11
  Select_04(1b) : Outport  Port=12
  Select_03(1b) : Outport  Port=13
  Select_02(1b) : Outport  Port=14
  Select_01(1b) : Outport  Port=15
  Select_00(1b) : Outport  Port=16
  wires:
  Logical Operator22/1 -> Logical Operator2/3, Logical Operator3/3, Logical Operator15/3, Logical Operator16/3, Logical Operator12/3, Logical Operator11/3, Logical Operator8/3, Logical Operator7/3
  Addr_0(1b)/1 -> Logical Operator23/1, Logical Operator/4, Logical Operator9/4, Logical Operator11/4, Logical Operator15/4, Logical Operator13/4, Logical Operator7/4, Logical Operator4/4, Logical Operator2/4
  Logical Operator23/1 -> Logical Operator1/4, Logical Operator16/4, Logical Operator14/4, Logical Operator12/4, Logical Operator10/4, Logical Operator8/4, Logical Operator6/4, Logical Operator3/4
  Logical Operator5/1 -> Logical Operator16/2, Logical Operator15/2, Logical Operator14/2, Logical Operator13/2, Logical Operator8/2, Logical Operator7/2, Logical Operator6/2, Logical Operator4/2
  Addr_3(1b)/1 -> Lo`gical Operator4/1, Logical Operator8/1, Logical Operator7/1, Logical Operator6/1, Logical Operator4/1, Logical Operator3/1, Logical Operator2/1, Logical Operator1/1, Logical Operator/1
  Addr_1(1b)/1 -> Logical Operator22/1, Logical Operator14/3, Logical Operator13/3, Logical Operator10/3, Logical Operator9/3, Logical Operator6/3, Logical Operator4/3, Logical Operator1/3, Logical Operator/3
  Addr_2(1b)/1 -> Logical Operator5/1, Logical Operator3/2, Logical Operator9/2, Logical Operator12/2, Logical Operator11/2, Logical Operator10/2, Logical Operator2/2, Logical Operator1/2, Logical Operator/2
  Lo`gical Operator4/1 -> Logical Operator16/1, Logical Operator15/1, Logical Operator14/1, Logical Operator13/1, Logical Operator12/1, Logical Operator11/1, Logical Operator10/1, Logical Operator9/1
  Logical Operator16/1 -> Select_00(1b)/1
  Logical Operator15/1 -> Select_01(1b)/1
  Logical Operator14/1 -> Select_02(1b)/1
  Logical Operator13/1 -> Select_03(1b)/1
  Logical Operator12/1 -> Select_04(1b)/1
  Logical Operator11/1 -> Select_05(1b)/1
  Logical Operator10/1 -> Select_06(1b)/1
  Logical Operator9/1 -> Select_07(1b)/1
  Logical Operator8/1 -> Select_08(1b)/1
  Logical Operator7/1 -> Select_09(1b)/1
  Logical Operator6/1 -> Select_10(1b)/1
  Logical Operator4/1 -> Select_11(1b)/1
  Logical Operator1/1 -> Select_14(1b)/1
  Logical Operator2/1 -> Select_13(1b)/1
  Logical Operator3/1 -> Select_12(1b)/1
  Logical Operator/1 -> Select_15(1b)/1

== system Garbage/Dec_16_Enbl ==
  note: Decoder_4_to_16_Enabled
  Enable(1b) : Inport
  Addr_3(1b) : Inport  Port=2
  Addr_2(1b) : Inport  Port=3
  Addr_1(1b) : Inport  Port=4
  Addr_0(1b) : Inport  Port=5
  Lo`gical Operator4 : Logic  Operator=NOT  IconShape=distinctive  AllPortsSameDT=off  OutDataTypeStr=boolean
  Logical Operator{,1..4,6..16} (16 alike) : Logic  Inputs=5  IconShape=distinctive  AllPortsSameDT=off  OutDataTypeStr=boolean
  Logical Operator22 : Logic  Operator=NOT  IconShape=distinctive  AllPortsSameDT=off  OutDataTypeStr=boolean
  Logical Operator23 : Logic  Operator=NOT  IconShape=distinctive  AllPortsSameDT=off  OutDataTypeStr=boolean
  Logical Operator5 : Logic  Operator=NOT  IconShape=distinctive  AllPortsSameDT=off  OutDataTypeStr=boolean
  Select_15(1b) : Outport
  Select_14(1b) : Outport  Port=2
  Select_13(1b) : Outport  Port=3
  Select_12(1b) : Outport  Port=4
  Select_11(1b) : Outport  Port=5
  Select_10(1b) : Outport  Port=6
  Select_09(1b) : Outport  Port=7
  Select_08(1b) : Outport  Port=8
  Select_07(1b) : Outport  Port=9
  Select_06(1b) : Outport  Port=10
  Select_05(1b) : Outport  Port=11
  Select_04(1b) : Outport  Port=12
  Select_03(1b) : Outport  Port=13
  Select_02(1b) : Outport  Port=14
  Select_01(1b) : Outport  Port=15
  Select_00(1b) : Outport  Port=16
  wires:
  Logical Operator22/1 -> Logical Operator2/4, Logical Operator3/4, Logical Operator7/4, Logical Operator8/4, Logical Operator11/4, Logical Operator12/4, Logical Operator15/4, Logical Operator16/4
  Addr_0(1b)/1 -> Logical Operator/5, Logical Operator2/5, Logical Operator4/5, Logical Operator7/5, Logical Operator9/5, Logical Operator11/5, Logical Operator13/5, Logical Operator15/5, Logical Operator23/1
  Logical Operator23/1 -> Logical Operator1/5, Logical Operator3/5, Logical Operator6/5, Logical Operator8/5, Logical Operator10/5, Logical Operator12/5, Logical Operator14/5, Logical Operator16/5
  Logical Operator5/1 -> Logical Operator4/3, Logical Operator6/3, Logical Operator7/3, Logical Operator8/3, Logical Operator13/3, Logical Operator14/3, Logical Operator15/3, Logical Operator16/3
  Addr_3(1b)/1 -> Logical Operator/2, Logical Operator1/2, Logical Operator2/2, Logical Operator3/2, Logical Operator4/2, Logical Operator6/2, Logical Operator7/2, Logical Operator8/2, Lo`gical Operator4/1
  Addr_1(1b)/1 -> Logical Operator/4, Logical Operator1/4, Logical Operator4/4, Logical Operator6/4, Logical Operator9/4, Logical Operator10/4, Logical Operator13/4, Logical Operator14/4, Logical Operator22/1
  Addr_2(1b)/1 -> Logical Operator/3, Logical Operator1/3, Logical Operator2/3, Logical Operator3/3, Logical Operator9/3, Logical Operator10/3, Logical Operator11/3, Logical Operator12/3, Logical Operator5/1
  Lo`gical Operator4/1 -> Logical Operator9/2, Logical Operator10/2, Logical Operator11/2, Logical Operator12/2, Logical Operator13/2, Logical Operator14/2, Logical Operator15/2, Logical Operator16/2
  Logical Operator16/1 -> Select_00(1b)/1
  Logical Operator15/1 -> Select_01(1b)/1
  Logical Operator14/1 -> Select_02(1b)/1
  Logical Operator13/1 -> Select_03(1b)/1
  Logical Operator12/1 -> Select_04(1b)/1
  Logical Operator11/1 -> Select_05(1b)/1
  Logical Operator10/1 -> Select_06(1b)/1
  Logical Operator9/1 -> Select_07(1b)/1
  Logical Operator8/1 -> Select_08(1b)/1
  Logical Operator7/1 -> Select_09(1b)/1
  Logical Operator6/1 -> Select_10(1b)/1
  Logical Operator4/1 -> Select_11(1b)/1
  Logical Operator1/1 -> Select_14(1b)/1
  Logical Operator2/1 -> Select_13(1b)/1
  Logical Operator3/1 -> Select_12(1b)/1
  Logical Operator/1 -> Select_15(1b)/1
  Enable(1b)/1 -> Logical Operator/1, Logical Operator1/1, Logical Operator2/1, Logical Operator3/1, Logical Operator4/1, Logical Operator6/1, Logical Operator7/1, Logical Operator8/1, Logical Operator9/1, Logical Operator10/1, Logical Operator11/1, Logical Operator12/1, Logical Operator13/1, Logical Operator14/1, Logical Operator15/1, Logical Operator16/1

== system Garbage/Dec_16_Enbl_Invr ==
  note: Decoder_4_to_16_Enabled_Inverting
  Enable(1b) : Inport
  Addr_3(1b) : Inport  Port=2
  Addr_2(1b) : Inport  Port=3
  Addr_1(1b) : Inport  Port=4
  Addr_0(1b) : Inport  Port=5
  Lo`gical Operator4 : Logic  Operator=NOT  IconShape=distinctive  AllPortsSameDT=off  OutDataTypeStr=boolean
  Logical Operator{,1..4,6..16} (16 alike) : Logic  Operator=NAND  Inputs=5  IconShape=distinctive  AllPortsSameDT=off  OutDataTypeStr=boolean
  Logical Operator22 : Logic  Operator=NOT  IconShape=distinctive  AllPortsSameDT=off  OutDataTypeStr=boolean
  Logical Operator23 : Logic  Operator=NOT  IconShape=distinctive  AllPortsSameDT=off  OutDataTypeStr=boolean
  Logical Operator5 : Logic  Operator=NOT  IconShape=distinctive  AllPortsSameDT=off  OutDataTypeStr=boolean
  Select_15(1b) : Outport
  Select_14(1b) : Outport  Port=2
  Select_13(1b) : Outport  Port=3
  Select_12(1b) : Outport  Port=4
  Select_11(1b) : Outport  Port=5
  Select_10(1b) : Outport  Port=6
  Select_09(1b) : Outport  Port=7
  Select_08(1b) : Outport  Port=8
  Select_07(1b) : Outport  Port=9
  Select_06(1b) : Outport  Port=10
  Select_05(1b) : Outport  Port=11
  Select_04(1b) : Outport  Port=12
  Select_03(1b) : Outport  Port=13
  Select_02(1b) : Outport  Port=14
  Select_01(1b) : Outport  Port=15
  Select_00(1b) : Outport  Port=16
  wires:
  Logical Operator22/1 -> Logical Operator2/4, Logical Operator3/4, Logical Operator7/4, Logical Operator8/4, Logical Operator11/4, Logical Operator12/4, Logical Operator15/4, Logical Operator16/4
  Addr_0(1b)/1 -> Logical Operator/5, Logical Operator2/5, Logical Operator4/5, Logical Operator7/5, Logical Operator9/5, Logical Operator11/5, Logical Operator13/5, Logical Operator15/5, Logical Operator23/1
  Logical Operator23/1 -> Logical Operator1/5, Logical Operator3/5, Logical Operator6/5, Logical Operator8/5, Logical Operator10/5, Logical Operator12/5, Logical Operator14/5, Logical Operator16/5
  Logical Operator5/1 -> Logical Operator4/3, Logical Operator6/3, Logical Operator7/3, Logical Operator8/3, Logical Operator13/3, Logical Operator14/3, Logical Operator15/3, Logical Operator16/3
  Addr_3(1b)/1 -> Logical Operator/2, Logical Operator1/2, Logical Operator2/2, Logical Operator3/2, Logical Operator4/2, Logical Operator6/2, Logical Operator7/2, Logical Operator8/2, Lo`gical Operator4/1
  Addr_1(1b)/1 -> Logical Operator/4, Logical Operator1/4, Logical Operator4/4, Logical Operator6/4, Logical Operator9/4, Logical Operator10/4, Logical Operator13/4, Logical Operator14/4, Logical Operator22/1
  Addr_2(1b)/1 -> Logical Operator/3, Logical Operator1/3, Logical Operator2/3, Logical Operator3/3, Logical Operator9/3, Logical Operator10/3, Logical Operator11/3, Logical Operator12/3, Logical Operator5/1
  Lo`gical Operator4/1 -> Logical Operator9/2, Logical Operator10/2, Logical Operator11/2, Logical Operator12/2, Logical Operator13/2, Logical Operator14/2, Logical Operator15/2, Logical Operator16/2
  Logical Operator16/1 -> Select_00(1b)/1
  Logical Operator15/1 -> Select_01(1b)/1
  Logical Operator14/1 -> Select_02(1b)/1
  Logical Operator13/1 -> Select_03(1b)/1
  Logical Operator12/1 -> Select_04(1b)/1
  Logical Operator11/1 -> Select_05(1b)/1
  Logical Operator10/1 -> Select_06(1b)/1
  Logical Operator9/1 -> Select_07(1b)/1
  Logical Operator8/1 -> Select_08(1b)/1
  Logical Operator7/1 -> Select_09(1b)/1
  Logical Operator6/1 -> Select_10(1b)/1
  Logical Operator4/1 -> Select_11(1b)/1
  Logical Operator1/1 -> Select_14(1b)/1
  Logical Operator2/1 -> Select_13(1b)/1
  Logical Operator3/1 -> Select_12(1b)/1
  Logical Operator/1 -> Select_15(1b)/1
  Enable(1b)/1 -> Logical Operator/1, Logical Operator1/1, Logical Operator2/1, Logical Operator3/1, Logical Operator4/1, Logical Operator6/1, Logical Operator7/1, Logical Operator8/1, Logical Operator9/1, Logical Operator10/1, Logical Operator11/1, Logical Operator12/1, Logical Operator13/1, Logical Operator14/1, Logical Operator15/1, Logical Operator16/1
